simulink model "TosAeb"  (R2021a)
settings: Solver=FixedStepDiscrete  StartTime=0.0  StopTime=inf  FixedStep=0.01  RelTol=1e-3
undefined symbols (not defined in the file or in its folder): ACCModSts, AccSts1, AEB_ObjectMovingOrientation, AEB_ObjectMovingState, CN_BTCActive, CN_CurrentGearR, CN_CurrentGearR_DHT, CN_PTCActive, CN_VDCActive, CN_VSC_EspOff, CN_VSC_FCW_AEB_Error, CN_VSC_FCW_AEB_NoDispay, CN_VSC_FCW_AEB_PerfmDgdtn, CN_VSC_FCW_AEB_TempUnAvlbl, CN_VSC_VehStandstill, CNaccelAvoidMax, CNaccelAvoidMin, CNbrakePedalPress, CNcomfortStrLeftAccelMax, CNcomfortStrLeftAccelMin, CNcomfortStrRightAccelMax, CNcomfortStrRightAccelMin, CNDrvDoorOpen, CNDrvModeEco, CNDrvModeNorm, CNDrvModeOther, CNDrvModeSport, CNDrvSBRreminder, CNNull, CNobjInvalidID, CNPassDoorOpen, CNPassSBRreminder, CNPEBSAccelNegInf, CNPEBSAccelNull, CNPEBSTimeInf, CNPEBSTimeNull, CNSTOPMAX, CNSTOPNULL, CNsubjWidth, CNSwtOFF, CNSwtON, CNTTCMAX, CNTTCNULL, CNVehConfigAEBCycNone, CNVehConfigAEBNone, CNVehConfigAEBPedNone, CNVehConfigFCWNone, CNVehConfigJANone, CollisionSeverity, FALSE, FCWSnvty, JACycObjCalcInfoDefaultParams, JAPedObjCalcInfoDefaultParams, JAVehObjCalcInfoDefaultParams, latCycObjCalcInfoDefaultParams, latPedObjCalcInfoDefaultParams, longObjCalcInfoDefaultParams, ModSts, movingStatus, ObjectType ... (+1112)
libraries: simulink x3031, AEBSFunctionLib x50, Ta_Library x33, AEBSStateMachineLib x23, Mylibrary x12, simulink_extras x7, ObjSelect_lib x3, dspstat3 x2
(part 76 of 102)

== system TosAeb/AEB_Objsel/TA_Processor/PreProcessor/ObjectPrecessor/TAObjInfoCalc/For Each Subsystem/Info_Check1/Basic_Info_check/HeadAngle/If Action Subsystem1/HeadAngle_c/Subsystem2 ==
  Obj_vy : Inport
  Obj_Vx : Inport  Port=2
  Atan2 : Trigonometry  Operator=atan2
  Constant : Constant  Value=pi/2
  MapToAngularInterval : Ta_Library/MapToAngularInterval  SystemSampleTime=-1  VariantActivationTime=update diagram  Latency=0  AutoFrameSizeCalculation=off
  Obj_Head : Outport
  wires:
  Constant/1 -> MapToAngularInterval/2
  Obj_Vx/1 -> Atan2/2
  Obj_vy/1 -> Atan2/1
  Atan2/1 -> MapToAngularInterval/1
  MapToAngularInterval/1 -> Obj_Head/1

== system TosAeb/AEB_Objsel/TA_Processor/PreProcessor/ObjectPrecessor/TAObjInfoCalc/For Each Subsystem/Info_Check1/Basic_Info_check/QuaCorrect/quality_recrectionelse/Quality_else/Factor_Cal ==
  dy_flg : Inport
  dx_flg : Inport  Port=2
  vx_flg : Inport  Port=3
  vy_flg : Inport  Port=4
  Add : Sum  Inputs=++++
  Constant : Constant  Value=5  OutDataTypeStr=single
  Data Type Conversion{,1..3} (4 alike) : DataTypeConversion  OutDataTypeStr=single
  Product : Product
  factor : Outport
  wires:
  Data Type Conversion1/1 -> Add/2
  Data Type Conversion3/1 -> Add/4
  vy_flg/1 -> Data Type Conversion3/1
  dy_flg/1 -> Data Type Conversion/1
  Data Type Conversion/1 -> Add/1
  Add/1 -> Product/1
  dx_flg/1 -> Data Type Conversion1/1
  vx_flg/1 -> Data Type Conversion2/1
  Data Type Conversion2/1 -> Add/3
  Product/1 -> factor/1
  Constant/1 -> Product/2

== system TosAeb/AEB_Objsel/TA_Processor/PreProcessor/ObjectPrecessor/TAObjInfoCalc/For Each Subsystem/Info_Check1/Basic_Info_check/QuaCorrect/quality_recrectionelse/Quality_else/Quality_Out ==
  Fus_TargetQuality : Inport
  factor : Inport  Port=2
  Add : Sum  Inputs=+-
  Data Type Conversion : DataTypeConversion  OutDataTypeStr=single
  Data Type Conversion1 : DataTypeConversion  OutDataTypeStr=uint8
  Fus_ObjQuality : Outport
  wires:
  Data Type Conversion1/1 -> Fus_ObjQuality/1
  Add/1 -> Data Type Conversion1/1
  factor/1 -> Add/2
  Data Type Conversion/1 -> Add/1
  Fus_TargetQuality/1 -> Data Type Conversion/1

== system TosAeb/AEB_Objsel/TA_Processor/PreProcessor/ObjectPrecessor/TAObjInfoCalc/For Each Subsystem/Info_Check1/Basic_Info_check/VxCorrect/Subsystem3/Subsystem2/Subsystem ==
  Fus_ObjVx_Single : Inport
  Fus_ObjDx_Single : Inport  Port=2
  Subsystem : Subsystem
  VbyDx : Subsystem
  VbyVx : Subsystem
  DisLong_flg : Outport
  wires:
  Subsystem/1(DisLong_flg) -> DisLong_flg/1
  VbyVx/1(VbyVx) -> Subsystem/2(VbyVx)
  VbyDx/1(VbyDx) -> Subsystem/1(VbyDx)
  Fus_ObjVx_Single/1 -> VbyVx/1(Fus_ObjVx_Single)
  Fus_ObjDx_Single/1 -> VbyDx/1(Fus_ObjDx_Single)

== system TosAeb/AEB_Objsel/TA_Processor/PreProcessor/ObjectPrecessor/TAObjInfoCalc/For Each Subsystem/Info_Check1/Basic_Info_check/VxCorrect/Subsystem3/Subsystem2/Subsystem1 ==
  Veh_AEBFunState : Inport
  Compare To Constant : simulink/Logic and Bit Operations/Compare To Constant  relop===  const=0  OutDataTypeStr=boolean
  AEB_State : Outport
  wires:
  Compare To Constant/1 -> AEB_State/1
  Veh_AEBFunState/1 -> Compare To Constant/1

== system TosAeb/AEB_Objsel/TA_Processor/PreProcessor/ObjectPrecessor/TAObjInfoCalc/For Each Subsystem/Info_Check1/Basic_Info_check/VyCorrect/Subsystem3/Subsystem2/Subsystem ==
  Fus_ObjVy_Single : Inport
  Fus_ObjDy_Single : Inport  Port=2
  Subsystem : Subsystem
  VbyDy : Subsystem
  VbyVy : Subsystem
  DisLat_flg : Outport
  wires:
  Fus_ObjDy_Single/1 -> VbyDy/1(Fus_ObjDy_Single)
  Fus_ObjVy_Single/1 -> VbyVy/1(Fus_ObjVy_Single)
  VbyDy/1(VbyDy) -> Subsystem/1(VbyDy)
  VbyVy/1(VbyVy) -> Subsystem/2(VbyVy)
  Subsystem/1(DisLat_flg) -> DisLat_flg/1

== system TosAeb/AEB_Objsel/TA_Processor/PreProcessor/ObjectPrecessor/TAObjInfoCalc/For Each Subsystem/Info_Check1/Basic_Info_check/VyCorrect/Subsystem3/Subsystem2/Subsystem1 ==
  Veh_AEBFunState : Inport
  Compare To Constant : simulink/Logic and Bit Operations/Compare To Constant  relop===  const=1  OutDataTypeStr=boolean
  AEB_State : Outport
  wires:
  Veh_AEBFunState/1 -> Compare To Constant/1
  Compare To Constant/1 -> AEB_State/1

== system TosAeb/AEB_Objsel/TA_Processor/PreProcessor/ObjectPrecessor/TAObjInfoCalc/For Each Subsystem/Info_Check1/Basic_Info_check/VyCorrect/Subsystem3/Subsystem2/Subsystem2 ==
  Fus_TargetState : Inport
  Veh_YawRate : Inport  Port=2
  Abs : Abs
  Compare To Constant : simulink/Logic and Bit Operations/Compare To Constant  relop=<=  const=0.02  OutDataTypeStr=boolean
  Compare To Constant1 : simulink/Logic and Bit Operations/Compare To Constant  relop===  const=2  OutDataTypeStr=boolean
  Compare To Constant2 : simulink/Logic and Bit Operations/Compare To Constant  relop===  const=3  OutDataTypeStr=boolean
  Logical Operator : Logic  Operator=OR  AllPortsSameDT=off  OutDataTypeStr=boolean
  Logical Operator1 : Logic  AllPortsSameDT=off  OutDataTypeStr=boolean
  State_flg : Outport
  wires:
  Logical Operator1/1 -> State_flg/1
  Logical Operator/1 -> Logical Operator1/1
  Compare To Constant2/1 -> Logical Operator/2
  Compare To Constant1/1 -> Logical Operator/1
  Fus_TargetState/1 -> Compare To Constant1/1, Compare To Constant2/1
  Veh_YawRate/1 -> Abs/1
  Abs/1 -> Compare To Constant/1
  Compare To Constant/1 -> Logical Operator1/2

== system TosAeb/FcwAebJa_Step_sys/ALG_AEBSystem/ALG_AEBController/AEB_FuncImpManagementApp/FuncManeuArbCalc/brkFuncManeuArbCalc/axTgtReqValueCalc1/AebfullTriggerInfoCalc/AEBTriggerRelCalc/AEBTriggerInfo/AEBTriggerInfo/dxSecureCalc/dxSecureCalc ==
  subjVel : Inport
  vxPredicted : Inport  Port=2
  Abs1 : Abs
  dxSecure Lookup Table : Lookup_n-D  BreakpointsForDimension1=P_AEB_FIM_dxSecureX1_vEgo  BreakpointsForDimension2=P_AEB_FIM_dxSecureX2_vRel  Table=P_AEB_FIM_dxSecureY  InternalRulePriority=Speed  RndMeth=Simplest  InputSameDT=on
      settings: InputPortMap=u0,u1
  dxSecure : Outport
  wires:
  subjVel/1 -> dxSecure Lookup Table/1
  vxPredicted/1 -> Abs1/1
  dxSecure Lookup Table/1 -> dxSecure/1
  Abs1/1 -> dxSecure Lookup Table/2

== system TosAeb/FcwAebJa_Step_sys/ALG_AEBSystem/ALG_AEBController/AEB_FuncImpManagementApp/FuncManeuArbCalc/brkFuncManeuArbCalc/axTgtReqValueCalc1/AebfullTriggerInfoCalc/Subsystem/ObjectPredict/If Action Subsystem/dxPredictedCalc ==
  tPredicted : Inport
  dxFrontBumper : Inport  Port=2
  vx : Inport  Port=3
  ax : Inport  Port=4
  Add : Sum  Inputs=+++
  Constant : Constant  Value=0.5  OutDataTypeStr=single
  Constant1 : Constant  Value=CNNull
  Max : MinMax  Function=max  Inputs=2
  Product{,1..3} (4 alike) : Product
  dxFrontBumperPredicted : Outport
  wires:
  Max/1 -> dxFrontBumperPredicted/1
  Constant1/1 -> Max/2
  Product1/1 -> Product3/2
  tPredicted/1 -> Product/1, Product1/2, Product1/1
  dxFrontBumper/1 -> Add/1
  Add/1 -> Max/1
  Constant/1 -> Product2/2
  Product2/1 -> Product3/1
  ax/1 -> Product2/1
  Product/1 -> Add/3
  vx/1 -> Product/2
  Product3/1 -> Add/2

== system TosAeb/FcwAebJa_Step_sys/ALG_AEBSystem/ALG_AEBController/AEB_FuncImpManagementApp/FuncManeuArbCalc/brkFuncManeuArbCalc/axTgtReqValueCalc1/AebfullTriggerInfoCalc/Subsystem/ObjectPredict/If Action Subsystem/dxPredictedCalc1 ==
  ax : Inport
  tPredicted : Inport  Port=2
  vx : Inport  Port=3
  subjVel : Inport  Port=4
  Add1 : Sum
  Add2 : Sum
  Compare To Constant : simulink/Logic and Bit Operations/Compare To Constant  relop=<=  const=CNNull  OutDataTypeStr=boolean
  Constant : Constant  Value=CNNull
  Product3 : Product
  Subtract : Sum  Inputs=+-
  Switch : Switch  Criteria=u2 ~= 0
  vxAbsObjPredicted : Outport
  vxPredicted : Outport  Port=2
  wires:
  subjVel/1 -> Add2/2, Subtract/2
  Add1/1 -> Add2/1  "vxPredicted"
  Switch/1 -> Subtract/1, vxAbsObjPredicted/1
  Constant/1 -> Switch/1
  Compare To Constant/1 -> Switch/2
  Subtract/1 -> vxPredicted/1
  Add2/1 -> Compare To Constant/1, Switch/3
  vx/1 -> Add1/2
  Product3/1 -> Add1/1
  tPredicted/1 -> Product3/2
  ax/1 -> Product3/1

== system TosAeb/FcwAebJa_Step_sys/ALG_AEBSystem/ALG_AEBController/AEB_FuncImpManagementApp/FuncManeuArbCalc/brkFuncManeuArbCalc/axTgtReqValueCalc1/AebfullTriggerInfoCalc/Subsystem/ObjectPredict/If Action Subsystem/tPredictedCalc ==
  Constant : Constant  Value=P_AEB_FIM_ObjectPredictTimeMax
  Constant1 : Constant  Value=0  OutDataTypeStr=single
  MinMax1 : MinMax  Function=max  Inputs=2
  tPredicted : Outport
  wires:
  MinMax1/1 -> tPredicted/1  "AEB_FIM_ObjectPredictTime4Control"
  Constant/1 -> MinMax1/1
  Constant1/1 -> MinMax1/2

== system TosAeb/FcwAebJa_Step_sys/ALG_AEBSystem/ALG_AEBController/AEB_FuncImpManagementApp/FuncManeuArbCalc/brkFuncManeuArbCalc/axTgtReqValueCalc1/axTgtReqValueCalc4AebControl/JerkFuncDecCalc/JerkFuncDecCalc/JerkFuncDecCalc/JerkFuncDecCalc/JerkFuncDecCalc ==
  aErroraDesiredaTgtReqValue : Inport
  JerkFuncDecFactor : Inport  Port=2
  JerkFuncDecK1 : Inport  Port=3
  JerkFuncDecMax : Inport  Port=4
  Add : Sum
  Compare To Constant : simulink/Logic and Bit Operations/Compare To Constant  relop=<  const=CNNull  OutDataTypeStr=boolean
  Constant : Constant  Value=P_AEB_FIM_DecJerkStep4DecControl
  Constant1 : Constant  Value=CNNull
  Max : MinMax  Function=max  Inputs=2
  Product : Product
  Switch1 : Switch  Criteria=u2 ~= 0
  JerkFuncDec : Outport
  wires:
  Constant1/1 -> Switch1/3
  Product/1 -> Switch1/1
  JerkFuncDecFactor/1 -> Product/1
  Constant/1 -> Product/2
  aErroraDesiredaTgtReqValue/1 -> Compare To Constant/1
  Add/1 -> Max/1
  JerkFuncDecK1/1 -> Add/2
  Compare To Constant/1 -> Switch1/2
  Switch1/1 -> Add/1
  JerkFuncDecMax/1 -> Max/2
  Max/1 -> JerkFuncDec/1

== system TosAeb/FcwAebJa_Step_sys/ALG_AEBSystem/ALG_AEBController/AEB_FuncImpManagementApp/FuncManeuArbCalc/brkFuncManeuArbCalc/axTgtReqValueCalc1/axTgtReqValueCalc4AebControl/JerkFuncDecCalc/JerkFuncDecCalc/JerkFuncDecCalc/JerkFuncDecCalc/JerkFuncDecFactor ==
  note: 设计思路：
      | 若期望减速度小于最大减速度，
      | 则全速追踪
      | 若望减速度大于最大减速度，且小于阈值
      | 则放大系数，快速追踪
  note: 小误差放大，快速追踪目标值
  DeltaaDesiredaTgtReqValue : Inport
  aDesired : Inport  Port=2
  accelMin : Inport  Port=3
  1-D Lookup Table : Lookup_n-D  NumberOfTableDimensions=1  BreakpointsForDimension1=P_AEB_FIM_DecJerkFactorX_ErroraDesiredaTgt  Table=P_AEB_FIM_DecJerkFactorY  ExtrapMethod=Clip  InternalRulePriority=Speed  RndMeth=Simplest  InputSameDT=on
  Compare To Constant : simulink/Logic and Bit Operations/Compare To Constant  relop=>  const=CNNull  OutDataTypeStr=boolean
  Compare To Constant1 : simulink/Logic and Bit Operations/Compare To Constant  relop=>  const=P_AEB_FIM_aErroraDesiredaMaxThres4FastDec  OutDataTypeStr=boolean
  Constant : Constant  Value=250
  Constant1 : Constant  Value=P_AEB_FIM_FastDecFactorGain
  Product : Product
  Subtract1 : Sum  Inputs=+-
  Switch : Switch  Criteria=u2 ~= 0
  Switch1 : Switch  Criteria=u2 ~= 0
  JerkFuncDecFactor : Outport
  wires:
  DeltaaDesiredaTgtReqValue/1 -> 1-D Lookup Table/1
  accelMin/1 -> Subtract1/1
  aDesired/1 -> Subtract1/2
  Subtract1/1 -> Compare To Constant/1, Compare To Constant1/1  "DeltaaDesiredaMin"
  Switch1/1 -> Switch/3
  Constant/1 -> Switch/1
  Compare To Constant/1 -> Switch/2
  Compare To Constant1/1 -> Switch1/2
  1-D Lookup Table/1 -> Switch1/3, Product/2
  Constant1/1 -> Product/1
  Switch/1 -> JerkFuncDecFactor/1
  Product/1 -> Switch1/1

== system TosAeb/FcwAebJa_Step_sys/ALG_AEBSystem/ALG_AEBController/AEB_FuncImpManagementApp/FuncManeuArbCalc/brkFuncManeuArbCalc/axTgtReqValueCalc1/axTgtReqValueCalc4AebControl/JerkFuncDecCalc/JerkFuncDecCalc/JerkFuncDecCalc/JerkFuncDecCalc/aErroraDesiredaTgtReqValue ==
  axTgtReqValueK1 : Inport
  aDesired : Inport  Port=2
  Subtract : Sum  Inputs=+-
  aErroraDesiredaTgtReqValue : Outport
  wires:
  Subtract/1 -> aErroraDesiredaTgtReqValue/1
  aDesired/1 -> Subtract/1
  axTgtReqValueK1/1 -> Subtract/2

== system TosAeb/FcwAebJa_Step_sys/ALG_AEBSystem/ALG_AEBController/AEB_FuncImpManagementApp/FuncManeuArbCalc/brkFuncManeuArbCalc/axTgtReqValueCalc1/axTgtReqValueCalc4AebControl/aDesiredCalc/If Action Subsystem1/accelAvoidLowLvlBrkCalc/Situation1 ==
  dxEgoBrake : Inport
  vx : Inport  Port=2
  tObjStop : Inport  Port=3
  subjVel : Inport  Port=4
  vxAbsObj : Inport  Port=5
  axAbsObj : Inport  Port=6
  function : TriggerPort  TriggerType=function-call  VariantControl=Variant
  Bus Creator : BusCreator  Inputs=6
  Bus Selector : BusSelector  OutputSignals=dxEgoBrake,vx,tObjStop
  Bus Selector1 : BusSelector  OutputSignals=axAbsObj,vx,dxEgoBrake
  Bus Selector2 : BusSelector  OutputSignals=subjVel,vxAbsObj,axAbsObj,dxEgoBrake
  Case1 : Subsystem  TreatAsAtomicUnit=on
  Case2 : Subsystem  TreatAsAtomicUnit=on
  If : If  IfExpression=u1
  Merge : Merge
  collisionFirstFlgCalc : Subsystem
  accelAvoidLowLvlBrk : Outport
  wires:
  collisionFirstFlgCalc/1(collisionFirstFlg) -> If/1
  Bus Selector/3 -> collisionFirstFlgCalc/3(tObjStop)  "<tObjStop>"
  Bus Selector/2 -> collisionFirstFlgCalc/2(vx)  "<vx>"
  Bus Selector/1 -> collisionFirstFlgCalc/1(dxEgoBrake)  "<dxEgoBrake>"
  Bus Selector2/4 -> Case2/4(dxEgoBrake)  "<dxEgoBrake>"
  Bus Selector2/3 -> Case2/3(axAbsObj)  "<axAbsObj>"
  Bus Selector2/2 -> Case2/2(vxAbsObj)  "<vxAbsObj>"
  Bus Selector2/1 -> Case2/1(subjVel)  "<subjVel>"
  Bus Selector1/3 -> Case1/3(dxEgoBrake)  "<dxEgoBrake>"
  Bus Selector1/2 -> Case1/2(vx)  "<vx>"
  Bus Selector1/1 -> Case1/1(axAbsObj)  "<axAbsObj>"
  Bus Creator/1 -> Bus Selector/1, Bus Selector1/1, Bus Selector2/1
  vxAbsObj/1 -> Bus Creator/5  "vxAbsObj"
  axAbsObj/1 -> Bus Creator/6  "axAbsObj"
  dxEgoBrake/1 -> Bus Creator/1  "dxEgoBrake"
  subjVel/1 -> Bus Creator/4  "subjVel"
  vx/1 -> Bus Creator/2  "vx"
  tObjStop/1 -> Bus Creator/3  "tObjStop"
  [CASE2] -> Case2/Action
  If/2 -> [CASE2]
  Case2/1(accelAvoid) -> Merge/2
  Case1/1(accelAvoid) -> Merge/1
  Merge/1 -> accelAvoidLowLvlBrk/1
  If/1 -> Case1/Action

== system TosAeb/FcwAebJa_Step_sys/ALG_AEBSystem/ALG_AEBController/AEB_FuncImpManagementApp/FuncManeuArbCalc/brkFuncManeuArbCalc/axTgtReqValueCalc1/axTgtReqValueCalc4AebControl/aDesiredCalc/If Action Subsystem1/accelAvoidLowLvlBrkCalc/Situation2 ==
  axAbsObj : Inport
  vx : Inport  Port=2
  dxEgoBrake : Inport  Port=3
  function : TriggerPort  TriggerType=function-call  VariantControl=Variant
  Divide : Product  Inputs=*/
  Gain : Gain  Gain=2
  Math Function : Math  Operator=square  SignedPower=on  SaturateOnIntegerOverflow=on
  Subtract : Sum  Inputs=+-
  accelAvoidLowLvlBrk : Outport
  wires:
  Divide/1 -> Subtract/2
  Subtract/1 -> accelAvoidLowLvlBrk/1
  Gain/1 -> Divide/2
  dxEgoBrake/1 -> Gain/1
  Math Function/1 -> Divide/1
  vx/1 -> Math Function/1
  axAbsObj/1 -> Subtract/1

== system TosAeb/FcwAebJa_Step_sys/ALG_AEBSystem/ALG_AEBController/AEB_FuncImpManagementApp/FuncManeuArbCalc/brkFuncManeuArbCalc/axTgtReqValueCalc1/axTgtReqValueCalc4AebControl/aDesiredCalc/If Action Subsystem1/accelAvoidLowLvlBrkCalc/Situation3 ==
  note: aEgo^2
      | aAvoid = ----------------------------------
      | 		vObj^2
      | 		---------  - 2(dRel - dScure)
      | 		aObj
  subjVel : Inport
  vxAbsObj : Inport  Port=2
  axAbsObj : Inport  Port=3
  dxEgoBrake : Inport  Port=4
  function : TriggerPort  TriggerType=function-call  VariantControl=Variant
  Divide : Product  Inputs=*/
  Divide1 : Product  Inputs=*/
  Gain : Gain  Gain=2
  Math Function : Math  Operator=square  SignedPower=on  SaturateOnIntegerOverflow=on
  Math Function1 : Math  Operator=square  SignedPower=on  SaturateOnIntegerOverflow=on
  Subtract : Sum  Inputs=+-
  accelAvoidLowLvlBrk : Outport
  wires:
  Subtract/1 -> Divide1/2
  dxEgoBrake/1 -> Gain/1
  axAbsObj/1 -> Divide/2
  Divide/1 -> Subtract/1
  Math Function1/1 -> Divide1/1
  Divide1/1 -> accelAvoidLowLvlBrk/1
  Gain/1 -> Subtract/2
  Math Function/1 -> Divide/1
  subjVel/1 -> Math Function1/1
  vxAbsObj/1 -> Math Function/1

== system TosAeb/FcwAebJa_Step_sys/ALG_AEBSystem/ALG_AEBController/AEB_FuncImpManagementApp/FuncManeuArbCalc/brkFuncManeuArbCalc/axTgtReqValueCalc1/axTgtReqValueCalc4AebControl/aDesiredCalc/If Action Subsystem1/accelAvoidLowLvlBrkCalc/Situation4 ==
  function : TriggerPort  TriggerType=function-call  VariantControl=Variant
  Constant : Constant  Value=CNNull  VectorParams1D=off
  accelAvoidLowLvlBrk : Outport
  wires:
  Constant/1 -> accelAvoidLowLvlBrk/1

== system TosAeb/FcwAebJa_Step_sys/ALG_AEBSystem/ALG_AEBController/AEB_FuncImpManagementApp/FuncManeuArbCalc/brkFuncManeuArbCalc/axTgtReqValueCalc1/axTgtReqValueCalc4AebControl/aDesiredCalc/If Action Subsystem1/accelAvoidLowLvlBrkCalc/accelAvoidLowLvlBrkLimit ==
  accelAvoidLowLvlBrkNoLimit : Inport
  Saturation : Saturate  UpperLimit=CNNull  LowerLimit=CNaccelAvoidMin
      settings: AttributesFormatString=|
      | UpperLimit:%<UpperLimit>
      | LowerLimit:%<LowerLimit>
  accelAvoidLowLvlBrk : Outport
  wires:
  Saturation/1 -> accelAvoidLowLvlBrk/1
  accelAvoidLowLvlBrkNoLimit/1 -> Saturation/1

== system TosAeb/FcwAebJa_Step_sys/ALG_AEBSystem/ALG_AEBController/AEB_FuncImpManagementApp/FuncManeuArbCalc/brkFuncManeuArbCalc/axTgtReqValueCalc1/axTgtReqValueCalc4AebControl/aDesiredCalc/If Action Subsystem1/tObjStopCalc/StopTimeFlow/tStopFuncCalc ==
  velRel : Inport
  accelRel : Inport  Port=2
  tStopFuncCalc : TriggerPort  TriggerType=function-call  VariantControl=Variant
      settings: CopyFcn=Stateflow.SLINSF.SimulinkMan.removeBlockPreDeleteFcn  PreDeleteFcn=Stateflow.SLINSF.SimulinkMan.unsafePredeleteFcn
  Abs : Abs
  Abs1 : Abs
  Divide : Product  Inputs=*/
  temptStop : Outport
  wires:
  accelRel/1 -> Abs1/1
  Divide/1 -> temptStop/1
  Abs1/1 -> Divide/2
  Abs/1 -> Divide/1
  velRel/1 -> Abs/1

== system TosAeb/FcwAebJa_Step_sys/ALG_AEBSystem/ALG_AEBController/AEB_FuncImpManagementApp/FuncManeuArbCalc/brkFuncManeuArbCalc/axTgtReqValueCalc1/axTgtReqValueCalc4AebControl/axTgtReqValueCalc/axTgtReqValueFinal/axTgtReqValueCalc/GradientOpt ==
  JerkFuncInc : Inport
  JerkFuncDec : Inport  Port=2
  Compare To Constant : simulink/Logic and Bit Operations/Compare To Constant  relop=<  const=0  OutDataTypeStr=boolean
  Compare To Constant1 : simulink/Logic and Bit Operations/Compare To Constant  relop=>  const=0  OutDataTypeStr=boolean
  Constant : Constant  Value=64  OutDataTypeStr=single
  Constant1 : Constant  Value=-64  OutDataTypeStr=single
  Switch : Switch  Criteria=u2 ~= 0
  Switch1 : Switch  Criteria=u2 ~= 0
  JerkFuncIncOpt : Outport
  JerkFuncDecOpt : Outport  Port=2
  wires:
  Switch1/1 -> JerkFuncDecOpt/1
  Switch/1 -> JerkFuncIncOpt/1
  Constant1/1 -> Switch1/1
  Compare To Constant1/1 -> Switch1/2
  JerkFuncDec/1 -> Compare To Constant1/1, Switch1/3
  Constant/1 -> Switch/1
  JerkFuncInc/1 -> Compare To Constant/1, Switch/3
  Compare To Constant/1 -> Switch/2

== system TosAeb/FcwAebJa_Step_sys/ALG_AEBSystem/ALG_AEBController/AEB_FuncImpManagementApp/FuncManeuArbCalc/brkFuncManeuArbCalc/axTgtReqValueCalc1/axTgtReqValueCalc4AebControl/axTgtReqValueCalc/axTgtReqValueFinal/axTgtReqValueCalc/JerkTimeSlot ==
  JerkFuncInc : Inport
  JerkFuncDec : Inport  Port=2
  Ts : Inport  Port=3
  aDesired : Inport  Port=4
  axTgtReqValueK1 : Inport  Port=5
  Product : Product
  Product1 : Product
  Subtract : Sum  Inputs=+-
  JerkIncTimeSlot : Outport
  JerkDecTimeSlot : Outport  Port=2
  aErrorADesiredAxTgtReq : Outport  Port=3
  wires:
  Subtract/1 -> aErrorADesiredAxTgtReq/1
  axTgtReqValueK1/1 -> Subtract/2
  aDesired/1 -> Subtract/1
  Product1/1 -> JerkDecTimeSlot/1
  Product/1 -> JerkIncTimeSlot/1
  JerkFuncDec/1 -> Product1/2
  Ts/1 -> Product1/1, Product/2
  JerkFuncInc/1 -> Product/1

== system TosAeb/FcwAebJa_Step_sys/ALG_AEBSystem/ALG_AEBController/AEB_FuncImpManagementApp/FuncManeuArbCalc/brkFuncManeuArbCalc/axTgtReqValueCalc1/axTgtReqValueCalc4AebControl/axTgtReqValueCalc/axTgtReqValueFinal/axTgtReqValueCalc/axTgtReqValueCalc ==
  JerkIncTimeSlot : Inport
  JerkDecTimeSlot : Inport  Port=2
  aErrorADesiredAxTgtReq : Inport  Port=3
  accelMax : Inport  Port=4
  accelMin : Inport  Port=5
  axTgtReqValueK1 : Inport  Port=6
  aDesired : Inport  Port=7
  axTgtReqValueFlow : Stateflow chart  TreatAsAtomicUnit=on
  axTgtReqValue : Outport
  wires:
  axTgtReqValueFlow/1(axTgtReqValue) -> axTgtReqValue/1
  axTgtReqValueK1/1 -> axTgtReqValueFlow/7(axTgtReqValueK1)
  accelMin/1 -> axTgtReqValueFlow/6(accelMin)
  accelMax/1 -> axTgtReqValueFlow/5(accelMax)
  aDesired/1 -> axTgtReqValueFlow/4(accelAvoid)
  aErrorADesiredAxTgtReq/1 -> axTgtReqValueFlow/3(aErrorADesiredAxTgtReq)
  JerkDecTimeSlot/1 -> axTgtReqValueFlow/2(JerkDecTimeSlot)
  JerkIncTimeSlot/1 -> axTgtReqValueFlow/1(JerkIncTimeSlot)

== system TosAeb/FcwAebJa_Step_sys/ALG_AEBSystem/ALG_AEBController/AEB_FuncImpManagementApp/FuncManeuArbCalc/brkFuncManeuArbCalc/axTgtReqValueCalc1/axTgtReqValueCalc4AebControl/axTgtReqValueCalc/axTgtReqValueFinal1/axTgtReqValueCalc/GradientOpt ==
  JerkFuncInc : Inport
  JerkFuncDec : Inport  Port=2
  Compare To Constant : simulink/Logic and Bit Operations/Compare To Constant  relop=<  const=0  OutDataTypeStr=boolean
  Compare To Constant1 : simulink/Logic and Bit Operations/Compare To Constant  relop=>  const=0  OutDataTypeStr=boolean
  Constant : Constant  Value=64  OutDataTypeStr=single
  Constant1 : Constant  Value=-64  OutDataTypeStr=single
  Switch : Switch  Criteria=u2 ~= 0
  Switch1 : Switch  Criteria=u2 ~= 0
  JerkFuncIncOpt : Outport
  JerkFuncDecOpt : Outport  Port=2
  wires:
  Switch1/1 -> JerkFuncDecOpt/1
  Switch/1 -> JerkFuncIncOpt/1
  Constant1/1 -> Switch1/1
  Compare To Constant1/1 -> Switch1/2
  JerkFuncDec/1 -> Compare To Constant1/1, Switch1/3
  Constant/1 -> Switch/1
  JerkFuncInc/1 -> Compare To Constant/1, Switch/3
  Compare To Constant/1 -> Switch/2

== system TosAeb/FcwAebJa_Step_sys/ALG_AEBSystem/ALG_AEBController/AEB_FuncImpManagementApp/FuncManeuArbCalc/brkFuncManeuArbCalc/axTgtReqValueCalc1/axTgtReqValueCalc4AebControl/axTgtReqValueCalc/axTgtReqValueFinal1/axTgtReqValueCalc/JerkTimeSlot ==
  JerkFuncInc : Inport
  JerkFuncDec : Inport  Port=2
  Ts : Inport  Port=3
  aDesired : Inport  Port=4
  axTgtReqValueK1 : Inport  Port=5
  Product : Product
  Product1 : Product
  Subtract : Sum  Inputs=+-
  JerkIncTimeSlot : Outport
  JerkDecTimeSlot : Outport  Port=2
  aErrorADesiredAxTgtReq : Outport  Port=3
  wires:
  Subtract/1 -> aErrorADesiredAxTgtReq/1
  axTgtReqValueK1/1 -> Subtract/2
  aDesired/1 -> Subtract/1
  Product1/1 -> JerkDecTimeSlot/1
  Product/1 -> JerkIncTimeSlot/1
  JerkFuncDec/1 -> Product1/2
  Ts/1 -> Product1/1, Product/2
  JerkFuncInc/1 -> Product/1

== system TosAeb/FcwAebJa_Step_sys/ALG_AEBSystem/ALG_AEBController/AEB_FuncImpManagementApp/FuncManeuArbCalc/brkFuncManeuArbCalc/axTgtReqValueCalc1/axTgtReqValueCalc4AebControl/axTgtReqValueCalc/axTgtReqValueFinal1/axTgtReqValueCalc/axTgtReqValueCalc ==
  JerkIncTimeSlot : Inport
  JerkDecTimeSlot : Inport  Port=2
  aErrorADesiredAxTgtReq : Inport  Port=3
  accelMax : Inport  Port=4
  accelMin : Inport  Port=5
  axTgtReqValueK1 : Inport  Port=6
  aDesired : Inport  Port=7
  axTgtReqValueFlow : Stateflow chart  TreatAsAtomicUnit=on
  axTgtReqValue : Outport
  wires:
  axTgtReqValueFlow/1(axTgtReqValue) -> axTgtReqValue/1
  axTgtReqValueK1/1 -> axTgtReqValueFlow/7(axTgtReqValueK1)
  accelMin/1 -> axTgtReqValueFlow/6(accelMin)
  accelMax/1 -> axTgtReqValueFlow/5(accelMax)
  aDesired/1 -> axTgtReqValueFlow/4(accelAvoid)
  aErrorADesiredAxTgtReq/1 -> axTgtReqValueFlow/3(aErrorADesiredAxTgtReq)
  JerkDecTimeSlot/1 -> axTgtReqValueFlow/2(JerkDecTimeSlot)
  JerkIncTimeSlot/1 -> axTgtReqValueFlow/1(JerkIncTimeSlot)

== system TosAeb/FcwAebJa_Step_sys/ALG_AEBSystem/ALG_AEBController/AEB_JaObjCalcApp/JAPedTgtInfoCalc/JAPedObjCalcInfoCalc/JAPedObjInfoCalc/ObjCrossFlgCal/CollisionProbCalc/Subsystem/ObjBoundingBox/ObjLLCoordinate ==
  inputSignals : Inport
  Add : Sum  Inputs=+-  OutDataTypeStr=single
  Add1 : Sum  OutDataTypeStr=single
  Bus Selector : BusSelector  OutputSignals=dxPreRefPointObj,ObjWidthCorr,sincosCoef.sinCoef
  Bus Selector1 : BusSelector  OutputSignals=dyPreRefPointObj,ObjWidthCorr,sincosCoef.cosCoef
  Gain : Gain  Gain=0.5  OutDataTypeStr=single
  Gain1 : Gain  Gain=0.5  OutDataTypeStr=single
  Product1 : Product  OutDataTypeStr=single
  Product3 : Product  OutDataTypeStr=single
  dxPre_LLObj : Outport
  dyPreLLObj : Outport  Port=2
  wires:
  Bus Selector1/1 -> Add1/1  "<dyPreRefPointObj>"
  Bus Selector1/3 -> Product3/2  "<cosCoef>"
  Bus Selector1/2 -> Product3/1  "<ObjWidthCorr>"
  Product3/1 -> Gain/1
  Add1/1 -> dyPreLLObj/1
  Add/1 -> dxPre_LLObj/1
  Bus Selector/1 -> Add/1  "<dxPreRefPointObj>"
  Product1/1 -> Gain1/1
  Bus Selector/3 -> Product1/2  "<sinCoef>"
  Bus Selector/2 -> Product1/1  "<ObjWidthCorr>"
  inputSignals/1 -> Bus Selector1/1, Bus Selector/1
  Gain/1 -> Add1/2
  Gain1/1 -> Add/2

== system TosAeb/FcwAebJa_Step_sys/ALG_AEBSystem/ALG_AEBController/AEB_JaObjCalcApp/JAPedTgtInfoCalc/JAPedObjCalcInfoCalc/JAPedObjInfoCalc/ObjCrossFlgCal/CollisionProbCalc/Subsystem/ObjBoundingBox/ObjLUCoordinate ==
  inputSignals : Inport
  Add : Sum  Inputs=++-  OutDataTypeStr=single
  Add1 : Sum  Inputs=+++  OutDataTypeStr=single
  Bus Selector : BusSelector  OutputSignals=dxPreRefPointObj,ObjLengthCorr,sincosCoef.cosCoef,ObjWidthCorr,sincosCoef.sinCoef
  Bus Selector1 : BusSelector  OutputSignals=dyPreRefPointObj,ObjLengthCorr,sincosCoef.sinCoef,ObjWidthCorr,sincosCoef.cosCoef
  Gain : Gain  Gain=0.5  OutDataTypeStr=single
  Gain1 : Gain  Gain=0.5  OutDataTypeStr=single
  Product{,1..3} (4 alike) : Product  OutDataTypeStr=single
  dxPreLUObj : Outport
  dyPreLUobj : Outport  Port=2
  wires:
  inputSignals/1 -> Bus Selector1/1, Bus Selector/1
  Bus Selector/2 -> Product/1  "<ObjLengthCorr>"
  Bus Selector/3 -> Product/2  "<cosCoef>"
  Bus Selector/4 -> Product1/1  "<ObjWidthCorr>"
  Bus Selector/5 -> Product1/2  "<sinCoef>"
  Product1/1 -> Gain1/1
  Bus Selector/1 -> Add/1  "<dxPreRefPointObj>"
  Product/1 -> Add/2
  Add/1 -> dxPreLUObj/1
  Add1/1 -> dyPreLUobj/1
  Product2/1 -> Add1/2
  Product3/1 -> Gain/1
  Bus Selector1/2 -> Product2/1  "<ObjLengthCorr>"
  Bus Selector1/4 -> Product3/1  "<ObjWidthCorr>"
  Bus Selector1/3 -> Product2/2  "<sinCoef>"
  Bus Selector1/5 -> Product3/2  "<cosCoef>"
  Bus Selector1/1 -> Add1/1  "<dyPreRefPointObj>"
  Gain/1 -> Add1/3
  Gain1/1 -> Add/3

== system TosAeb/FcwAebJa_Step_sys/ALG_AEBSystem/ALG_AEBController/AEB_JaObjCalcApp/JAPedTgtInfoCalc/JAPedObjCalcInfoCalc/JAPedObjInfoCalc/ObjCrossFlgCal/CollisionProbCalc/Subsystem/ObjBoundingBox/ObjRLCoordinate ==
  inputSignals : Inport
  Add : Sum  OutDataTypeStr=single
  Add1 : Sum  Inputs=+-  OutDataTypeStr=single
  Bus Selector : BusSelector  OutputSignals=dxPreRefPointObj,ObjWidthCorr,sincosCoef.sinCoef
  Bus Selector1 : BusSelector  OutputSignals=dyPreRefPointObj,ObjWidthCorr,sincosCoef.cosCoef
  Gain : Gain  Gain=0.5  OutDataTypeStr=single
  Gain1 : Gain  Gain=0.5  OutDataTypeStr=single
  Product1 : Product  OutDataTypeStr=single
  Product3 : Product  OutDataTypeStr=single
  dxPreRLObj : Outport
  dyPreRLObj : Outport  Port=2
  wires:
  Bus Selector1/1 -> Add1/1  "<dyPreRefPointObj>"
  Bus Selector1/3 -> Product3/2  "<cosCoef>"
  Bus Selector1/2 -> Product3/1  "<ObjWidthCorr>"
  Product3/1 -> Gain1/1
  Add1/1 -> dyPreRLObj/1
  Add/1 -> dxPreRLObj/1
  Bus Selector/1 -> Add/1  "<dxPreRefPointObj>"
  Product1/1 -> Gain/1
  Bus Selector/3 -> Product1/2  "<sinCoef>"
  Bus Selector/2 -> Product1/1  "<ObjWidthCorr>"
  inputSignals/1 -> Bus Selector1/1, Bus Selector/1
  Gain/1 -> Add/2
  Gain1/1 -> Add1/2

== system TosAeb/FcwAebJa_Step_sys/ALG_AEBSystem/ALG_AEBController/AEB_JaObjCalcApp/JAPedTgtInfoCalc/JAPedObjCalcInfoCalc/JAPedObjInfoCalc/ObjCrossFlgCal/CollisionProbCalc/Subsystem/ObjBoundingBox/ObjRUCoordinate ==
  inputSignals : Inport
  Add : Sum  Inputs=+++  OutDataTypeStr=single
  Add1 : Sum  Inputs=++-  OutDataTypeStr=single
  Bus Selector : BusSelector  OutputSignals=dxPreRefPointObj,ObjLengthCorr,sincosCoef.cosCoef,ObjWidthCorr,sincosCoef.sinCoef
  Bus Selector1 : BusSelector  OutputSignals=dyPreRefPointObj,ObjLengthCorr,sincosCoef.sinCoef,ObjWidthCorr,sincosCoef.cosCoef
  Gain : Gain  Gain=0.5  OutDataTypeStr=single
  Gain1 : Gain  Gain=0.5  OutDataTypeStr=single
  Product{,1..3} (4 alike) : Product  OutDataTypeStr=single
  dxPreRUObj : Outport
  dyPreRUObj : Outport  Port=2
  wires:
  inputSignals/1 -> Bus Selector1/1, Bus Selector/1
  Bus Selector/2 -> Product/1  "<ObjLengthCorr>"
  Bus Selector/3 -> Product/2  "<cosCoef>"
  Bus Selector/4 -> Product1/1  "<ObjWidthCorr>"
  Bus Selector/5 -> Product1/2  "<sinCoef>"
  Product1/1 -> Gain1/1
  Bus Selector/1 -> Add/1  "<dxPreRefPointObj>"
  Product/1 -> Add/2
  Add/1 -> dxPreRUObj/1
  Add1/1 -> dyPreRUObj/1
  Product2/1 -> Add1/2
  Product3/1 -> Gain/1
  Bus Selector1/2 -> Product2/1  "<ObjLengthCorr>"
  Bus Selector1/4 -> Product3/1  "<ObjWidthCorr>"
  Bus Selector1/3 -> Product2/2  "<sinCoef>"
  Bus Selector1/5 -> Product3/2  "<cosCoef>"
  Bus Selector1/1 -> Add1/1  "<dyPreRefPointObj>"
  Gain/1 -> Add1/3
  Gain1/1 -> Add/3

== system TosAeb/FcwAebJa_Step_sys/ALG_AEBSystem/ALG_AEBController/AEB_JaObjCalcApp/JAPedTgtInfoCalc/JAPedObjCalcInfoCalc/JAPedObjInfoCalc/ObjCrossFlgCal/CollisionProbCalc/Subsystem/PreCrossFlgCalcFlow/VectorCrossAlgorithm/If Action Subsystem ==
  Action Port : ActionPort  ActionPortLabel=if(u1)
  Constant : Constant  Value=0  OutDataTypeStr=boolean
  CrossFlg : Outport
  wires:
  Constant/1 -> CrossFlg/1

== system TosAeb/FcwAebJa_Step_sys/ALG_AEBSystem/ALG_AEBController/AEB_JaObjCalcApp/JAPedTgtInfoCalc/JAPedObjCalcInfoCalc/JAPedObjInfoCalc/ObjCrossFlgCal/CollisionProbCalc/Subsystem/PreCrossFlgCalcFlow/VectorCrossAlgorithm/If Action Subsystem1 ==
  vectorMulit : Inport
  Coordinations : Inport  Port=2
  Action Port : ActionPort  ActionPortLabel=else
  Bus Selector : BusSelector  OutputSignals=m2,m1,y1,n1,n2,x1
  Bus Selector1 : BusSelector  OutputSignals=x2,x1,y1,n1,y2,m1
  Subsystem : Subsystem
  t : Subsystem
  u : Subsystem
  CrossFlg : Outport
  wires:
  Coordinations/1 -> Bus Selector1/1, Bus Selector/1
  Bus Selector/2 -> t/2(m1)  "<m1>"
  Bus Selector/3 -> t/3(y1)  "<y1>"
  Bus Selector/1 -> t/1(m2)  "<m2>"
  Bus Selector/4 -> t/4(n1)  "<n1>"
  Bus Selector/5 -> t/5(n2)  "<n2>"
  Bus Selector/6 -> t/6(x1)  "<x1>"
  Bus Selector1/3 -> u/3(y1)  "<y1>"
  Bus Selector1/2 -> u/2(x1)  "<x1>"
  Bus Selector1/4 -> u/4(n1)  "<n1>"
  Bus Selector1/1 -> u/1(x2)  "<x2>"
  Bus Selector1/5 -> u/5(y2)  "<y2>"
  Bus Selector1/6 -> u/6(m1)  "<m1>"
  t/1(temp_t) -> Subsystem/1(temp_u)
  u/1(temp_u) -> Subsystem/2(temp_t)
  Subsystem/1(CrossFlg) -> CrossFlg/1
  vectorMulit/1 -> Subsystem/3(vectorMulit)

== system TosAeb/FcwAebJa_Step_sys/ALG_AEBSystem/ALG_AEBController/AEB_JaObjCalcApp/JAPedTgtInfoCalc/JAPedObjCalcInfoCalc/JAPedObjInfoCalc/ObjCrossFlgCal/CollisionProbCalc/Subsystem/PreCrossFlgCalcFlow/VectorCrossAlgorithm/VectorMulit ==
  x1 : Inport  OutDataTypeStr=single
  x2 : Inport  Port=2  OutDataTypeStr=single
  n2 : Inport  Port=3  OutDataTypeStr=single
  n1 : Inport  Port=4  OutDataTypeStr=single
  y2 : Inport  Port=5  OutDataTypeStr=single
  y1 : Inport  Port=6  OutDataTypeStr=single
  m2 : Inport  Port=7  OutDataTypeStr=single
  m1 : Inport  Port=8  OutDataTypeStr=single
  Product : Product  OutDataTypeStr=single
  Product1 : Product  OutDataTypeStr=single
  Subtract{1..4} (4 alike) : Sum  Inputs=+-  OutDataTypeStr=single
  Subtract5 : Sum  Inputs=+-
  vectorMulit : Outport
  wires:
  x1/1 -> Subtract2/2
  x2/1 -> Subtract2/1
  Subtract2/1 -> Product/1
  Subtract3/1 -> Product/2
  y2/1 -> Subtract4/1
  y1/1 -> Subtract4/2
  m2/1 -> Subtract1/1
  m1/1 -> Subtract1/2
  Subtract4/1 -> Product1/1
  Subtract1/1 -> Product1/2
  Product/1 -> Subtract5/1
  Product1/1 -> Subtract5/2
  Subtract5/1 -> vectorMulit/1
  n2/1 -> Subtract3/1
  n1/1 -> Subtract3/2

== system TosAeb/FcwAebJa_Step_sys/ALG_AEBSystem/ALG_AEBController/AEB_JaObjCalcApp/JAPedTgtInfoCalc/JAPedObjCalcInfoCalc/JAPedObjInfoCalc/ObjCrossFlgCal/CollisionProbCalc/Subsystem/SubjBoundingBox/SubjLLCoordinate ==
  inputSignals : Inport
  Add2 : Sum  Inputs=+--  OutDataTypeStr=single
  Add3 : Sum  Inputs=+-+  OutDataTypeStr=single
  Bus Selector2 : BusSelector  OutputSignals=dxPreRefPoint,dxRearBumperToRearAxis,sincosCoef.cosCoef,subjWidth,sincosCoef.sinCoef
  Bus Selector3 : BusSelector  OutputSignals=dyPreRefPoint,dxRearBumperToRearAxis,sincosCoef.sinCoef,subjWidth,sincosCoef.cosCoef
  Gain2 : Gain  Gain=0.5  OutDataTypeStr=single
  Gain3 : Gain  Gain=0.5  OutDataTypeStr=single
  Product{4..7} (4 alike) : Product  OutDataTypeStr=single
  dxPreLL : Outport
  dyPreLL : Outport  Port=2
  wires:
  Gain3/1 -> Add3/3
  Bus Selector3/3 -> Product6/2  "<sinCoef>"
  Add3/1 -> dyPreLL/1
  Product7/1 -> Gain3/1
  Product6/1 -> Add3/2
  Product4/1 -> Add2/2
  Bus Selector3/5 -> Product7/2  "<cosCoef>"
  Product5/1 -> Gain2/1
  Gain2/1 -> Add2/3
  Add2/1 -> dxPreLL/1
  Bus Selector3/4 -> Product7/1  "<subjWidth>"
  Bus Selector3/2 -> Product6/1  "<dxRearBumperToRearAxis>"
  Bus Selector3/1 -> Add3/1  "<dyPreRefPoint>"
  inputSignals/1 -> Bus Selector3/1, Bus Selector2/1
  Bus Selector2/4 -> Product5/1  "<subjWidth>"
  Bus Selector2/2 -> Product4/1  "<dxRearBumperToRearAxis>"
  Bus Selector2/3 -> Product4/2  "<cosCoef>"
  Bus Selector2/1 -> Add2/1  "<dxPreRefPoint>"
  Bus Selector2/5 -> Product5/2  "<sinCoef>"

== system TosAeb/FcwAebJa_Step_sys/ALG_AEBSystem/ALG_AEBController/AEB_JaObjCalcApp/JAPedTgtInfoCalc/JAPedObjCalcInfoCalc/JAPedObjInfoCalc/ObjCrossFlgCal/CollisionProbCalc/Subsystem/SubjBoundingBox/SubjLUCoordinate ==
  inputSignals : Inport
  Add : Sum  Inputs=++-  OutDataTypeStr=single
  Add1 : Sum  Inputs=+++  OutDataTypeStr=single
  Bus Selector : BusSelector  OutputSignals=dxPreRefPoint,dxFrontBumperToRearAxis,sincosCoef.cosCoef,subjWidth,sincosCoef.sinCoef
  Bus Selector1 : BusSelector  OutputSignals=dyPreRefPoint,dxFrontBumperToRearAxis,sincosCoef.sinCoef,subjWidth,sincosCoef.cosCoef
  Gain : Gain  Gain=0.5  OutDataTypeStr=single
  Gain1 : Gain  Gain=0.5  OutDataTypeStr=single
  Product{,1..3} (4 alike) : Product  OutDataTypeStr=single
  dxPreLU : Outport
  dyPreLU : Outport  Port=2
  wires:
  Bus Selector/4 -> Product1/1  "<subjWidth>"
  Product3/1 -> Gain1/1
  Add/1 -> dxPreLU/1
  Bus Selector1/1 -> Add1/1  "<dyPreRefPoint>"
  Gain1/1 -> Add1/3
  Bus Selector1/4 -> Product3/1  "<subjWidth>"
  Product2/1 -> Add1/2
  Bus Selector/3 -> Product/2  "<cosCoef>"
  Add1/1 -> dyPreLU/1
  Bus Selector/5 -> Product1/2  "<sinCoef>"
  Bus Selector/2 -> Product/1  "<dxFrontBumperToRearAxis>"
  Bus Selector1/2 -> Product2/1  "<dxFrontBumperToRearAxis>"
  inputSignals/1 -> Bus Selector1/1, Bus Selector/1
  Bus Selector1/5 -> Product3/2  "<cosCoef>"
  Bus Selector/1 -> Add/1  "<dxPreRefPoint>"
  Gain/1 -> Add/3
  Product1/1 -> Gain/1
  Product/1 -> Add/2
  Bus Selector1/3 -> Product2/2  "<sinCoef>"

== system TosAeb/FcwAebJa_Step_sys/ALG_AEBSystem/ALG_AEBController/AEB_JaObjCalcApp/JAPedTgtInfoCalc/JAPedObjCalcInfoCalc/JAPedObjInfoCalc/ObjCrossFlgCal/CollisionProbCalc/Subsystem/SubjBoundingBox/SubjRLCoordinate ==
  inputSignals : Inport
  Add6 : Sum  Inputs=+-+  OutDataTypeStr=single
  Add7 : Sum  Inputs=+--  OutDataTypeStr=single
  Bus Selector6 : BusSelector  OutputSignals=dxPreRefPoint,dxRearBumperToRearAxis,sincosCoef.cosCoef,subjWidth,sincosCoef.sinCoef
  Bus Selector7 : BusSelector  OutputSignals=dyPreRefPoint,dxRearBumperToRearAxis,sincosCoef.sinCoef,subjWidth,sincosCoef.cosCoef
  Gain6 : Gain  Gain=0.5  OutDataTypeStr=single
  Gain7 : Gain  Gain=0.5  OutDataTypeStr=single
  Product{12..15} (4 alike) : Product  OutDataTypeStr=single
  dxPreRL : Outport
  dyPreRL : Outport  Port=2
  wires:
  Product12/1 -> Add6/2
  Gain6/1 -> Add6/3
  Product13/1 -> Gain6/1
  Add6/1 -> dxPreRL/1
  Bus Selector6/4 -> Product13/1  "<subjWidth>"
  Bus Selector6/5 -> Product13/2  "<sinCoef>"
  Bus Selector6/1 -> Add6/1  "<dxPreRefPoint>"
  Bus Selector6/2 -> Product12/1  "<dxRearBumperToRearAxis>"
  Bus Selector6/3 -> Product12/2  "<cosCoef>"
  inputSignals/1 -> Bus Selector7/1, Bus Selector6/1
  Add7/1 -> dyPreRL/1
  Product14/1 -> Add7/2
  Gain7/1 -> Add7/3
  Bus Selector7/1 -> Add7/1  "<dyPreRefPoint>"
  Bus Selector7/2 -> Product14/1  "<dxRearBumperToRearAxis>"
  Bus Selector7/4 -> Product15/1  "<subjWidth>"
  Product15/1 -> Gain7/1
  Bus Selector7/5 -> Product15/2  "<cosCoef>"
  Bus Selector7/3 -> Product14/2  "<sinCoef>"

== system TosAeb/FcwAebJa_Step_sys/ALG_AEBSystem/ALG_AEBController/AEB_JaObjCalcApp/JAPedTgtInfoCalc/JAPedObjCalcInfoCalc/JAPedObjInfoCalc/ObjCrossFlgCal/CollisionProbCalc/Subsystem/SubjBoundingBox/SubjRUCoordinate ==
  inputSignals : Inport
  Add4 : Sum  Inputs=+++  OutDataTypeStr=single
  Add5 : Sum  Inputs=++-  OutDataTypeStr=single
  Bus Selector4 : BusSelector  OutputSignals=dxPreRefPoint,dxFrontBumperToRearAxis,sincosCoef.cosCoef,subjWidth,sincosCoef.sinCoef
  Bus Selector5 : BusSelector  OutputSignals=dyPreRefPoint,dxFrontBumperToRearAxis,sincosCoef.sinCoef,subjWidth,sincosCoef.cosCoef
  Gain4 : Gain  Gain=0.5  OutDataTypeStr=single
  Gain5 : Gain  Gain=0.5  OutDataTypeStr=single
  Product{8..11} (4 alike) : Product  OutDataTypeStr=single
  dxPreRU : Outport
  dyPreRU : Outport  Port=2
  wires:
  Bus Selector4/4 -> Product9/1  "<subjWidth>"
  Bus Selector4/5 -> Product9/2  "<sinCoef>"
  Bus Selector4/3 -> Product8/2  "<cosCoef>"
  Bus Selector4/1 -> Add4/1  "<dxPreRefPoint>"
  inputSignals/1 -> Bus Selector5/1, Bus Selector4/1
  Bus Selector4/2 -> Product8/1  "<dxFrontBumperToRearAxis>"
  Product9/1 -> Gain4/1
  Bus Selector5/4 -> Product11/1  "<subjWidth>"
  Product10/1 -> Add5/2
  Product11/1 -> Gain5/1
  Add5/1 -> dyPreRU/1
  Gain5/1 -> Add5/3
  Bus Selector5/2 -> Product10/1  "<dxFrontBumperToRearAxis>"
  Bus Selector5/3 -> Product10/2  "<sinCoef>"
  Bus Selector5/5 -> Product11/2  "<cosCoef>"
  Add4/1 -> dxPreRU/1
  Gain4/1 -> Add4/3
  Product8/1 -> Add4/2
  Bus Selector5/1 -> Add5/1  "<dyPreRefPoint>"

== system TosAeb/FcwAebJa_Step_sys/ALG_AEBSystem/ALG_AEBController/AEB_JaObjCalcApp/JAVehTgtInfoCalc/AEBJAVehObjCalc/JAVehObjInfoCalc/ObjCrossFlgCal/CollisionProbCalc/Subsystem/ObjBoundingBoxRear/ObjLLCoordinate ==
  inputSignals : Inport
  Add : Sum  Inputs=+-  OutDataTypeStr=single
  Add1 : Sum  OutDataTypeStr=single
  Bus Selector : BusSelector  OutputSignals=dxPreRefPointObj,ObjWidthCorr,sincosCoef.sinCoef
  Bus Selector1 : BusSelector  OutputSignals=dyPreRefPointObj,ObjWidthCorr,sincosCoef.cosCoef
  Gain : Gain  Gain=0.5  OutDataTypeStr=single
  Gain1 : Gain  Gain=0.5  OutDataTypeStr=single
  Product1 : Product  OutDataTypeStr=single
  Product3 : Product  OutDataTypeStr=single
  dxPre_LLObj : Outport
  dyPreLLObj : Outport  Port=2
  wires:
  Bus Selector1/1 -> Add1/1  "<dyPreRefPointObj>"
  Bus Selector1/3 -> Product3/2  "<cosCoef>"
  Bus Selector1/2 -> Product3/1  "<ObjWidthCorr>"
  Product3/1 -> Gain/1
  Add1/1 -> dyPreLLObj/1
  Add/1 -> dxPre_LLObj/1
  Bus Selector/1 -> Add/1  "<dxPreRefPointObj>"
  Product1/1 -> Gain1/1
  Bus Selector/3 -> Product1/2  "<sinCoef>"
  Bus Selector/2 -> Product1/1  "<ObjWidthCorr>"
  inputSignals/1 -> Bus Selector1/1, Bus Selector/1
  Gain/1 -> Add1/2
  Gain1/1 -> Add/2

== system TosAeb/FcwAebJa_Step_sys/ALG_AEBSystem/ALG_AEBController/AEB_JaObjCalcApp/JAVehTgtInfoCalc/AEBJAVehObjCalc/JAVehObjInfoCalc/ObjCrossFlgCal/CollisionProbCalc/Subsystem/ObjBoundingBoxRear/ObjLUCoordinate ==
  inputSignals : Inport
  Add : Sum  Inputs=++-  OutDataTypeStr=single
  Add1 : Sum  Inputs=+++  OutDataTypeStr=single
  Bus Selector : BusSelector  OutputSignals=dxPreRefPointObj,ObjLengthCorr,sincosCoef.cosCoef,ObjWidthCorr,sincosCoef.sinCoef
  Bus Selector1 : BusSelector  OutputSignals=dyPreRefPointObj,ObjLengthCorr,sincosCoef.sinCoef,ObjWidthCorr,sincosCoef.cosCoef
  Gain : Gain  Gain=0.5  OutDataTypeStr=single
  Gain1 : Gain  Gain=0.5  OutDataTypeStr=single
  Product{,1..3} (4 alike) : Product  OutDataTypeStr=single
  dxPreLUObj : Outport
  dyPreLUobj : Outport  Port=2
  wires:
  inputSignals/1 -> Bus Selector1/1, Bus Selector/1
  Bus Selector/2 -> Product/1  "<ObjLengthCorr>"
  Bus Selector/3 -> Product/2  "<cosCoef>"
  Bus Selector/4 -> Product1/1  "<ObjWidthCorr>"
  Bus Selector/5 -> Product1/2  "<sinCoef>"
  Product1/1 -> Gain1/1
  Bus Selector/1 -> Add/1  "<dxPreRefPointObj>"
  Product/1 -> Add/2
  Add/1 -> dxPreLUObj/1
  Add1/1 -> dyPreLUobj/1
  Product2/1 -> Add1/2
  Product3/1 -> Gain/1
  Bus Selector1/2 -> Product2/1  "<ObjLengthCorr>"
  Bus Selector1/4 -> Product3/1  "<ObjWidthCorr>"
  Bus Selector1/3 -> Product2/2  "<sinCoef>"
  Bus Selector1/5 -> Product3/2  "<cosCoef>"
  Bus Selector1/1 -> Add1/1  "<dyPreRefPointObj>"
  Gain/1 -> Add1/3
  Gain1/1 -> Add/3

== system TosAeb/FcwAebJa_Step_sys/ALG_AEBSystem/ALG_AEBController/AEB_JaObjCalcApp/JAVehTgtInfoCalc/AEBJAVehObjCalc/JAVehObjInfoCalc/ObjCrossFlgCal/CollisionProbCalc/Subsystem/ObjBoundingBoxRear/ObjRLCoordinate ==
  inputSignals : Inport
  Add : Sum  OutDataTypeStr=single
  Add1 : Sum  Inputs=+-  OutDataTypeStr=single
  Bus Selector : BusSelector  OutputSignals=dxPreRefPointObj,ObjWidthCorr,sincosCoef.sinCoef
  Bus Selector1 : BusSelector  OutputSignals=dyPreRefPointObj,ObjWidthCorr,sincosCoef.cosCoef
  Gain : Gain  Gain=0.5  OutDataTypeStr=single
  Gain1 : Gain  Gain=0.5  OutDataTypeStr=single
  Product1 : Product  OutDataTypeStr=single
  Product3 : Product  OutDataTypeStr=single
  dxPreRLObj : Outport
  dyPreRLObj : Outport  Port=2
  wires:
  Bus Selector1/1 -> Add1/1  "<dyPreRefPointObj>"
  Bus Selector1/3 -> Product3/2  "<cosCoef>"
  Bus Selector1/2 -> Product3/1  "<ObjWidthCorr>"
  Product3/1 -> Gain1/1
  Add1/1 -> dyPreRLObj/1
  Add/1 -> dxPreRLObj/1
  Bus Selector/1 -> Add/1  "<dxPreRefPointObj>"
  Product1/1 -> Gain/1
  Bus Selector/3 -> Product1/2  "<sinCoef>"
  Bus Selector/2 -> Product1/1  "<ObjWidthCorr>"
  inputSignals/1 -> Bus Selector1/1, Bus Selector/1
  Gain/1 -> Add/2
  Gain1/1 -> Add1/2

== system TosAeb/FcwAebJa_Step_sys/ALG_AEBSystem/ALG_AEBController/AEB_JaObjCalcApp/JAVehTgtInfoCalc/AEBJAVehObjCalc/JAVehObjInfoCalc/ObjCrossFlgCal/CollisionProbCalc/Subsystem/ObjBoundingBoxRear/ObjRUCoordinate ==
  inputSignals : Inport
  Add : Sum  Inputs=+++  OutDataTypeStr=single
  Add1 : Sum  Inputs=++-  OutDataTypeStr=single
  Bus Selector : BusSelector  OutputSignals=dxPreRefPointObj,ObjLengthCorr,sincosCoef.cosCoef,ObjWidthCorr,sincosCoef.sinCoef
  Bus Selector1 : BusSelector  OutputSignals=dyPreRefPointObj,ObjLengthCorr,sincosCoef.sinCoef,ObjWidthCorr,sincosCoef.cosCoef
  Gain : Gain  Gain=0.5  OutDataTypeStr=single
  Gain1 : Gain  Gain=0.5  OutDataTypeStr=single
  Product{,1..3} (4 alike) : Product  OutDataTypeStr=single
  dxPreRUObj : Outport
  dyPreRUObj : Outport  Port=2
  wires:
  inputSignals/1 -> Bus Selector1/1, Bus Selector/1
  Bus Selector/2 -> Product/1  "<ObjLengthCorr>"
  Bus Selector/3 -> Product/2  "<cosCoef>"
  Bus Selector/4 -> Product1/1  "<ObjWidthCorr>"
  Bus Selector/5 -> Product1/2  "<sinCoef>"
  Product1/1 -> Gain1/1
  Bus Selector/1 -> Add/1  "<dxPreRefPointObj>"
  Product/1 -> Add/2
  Add/1 -> dxPreRUObj/1
  Add1/1 -> dyPreRUObj/1
  Product2/1 -> Add1/2
  Product3/1 -> Gain/1
  Bus Selector1/2 -> Product2/1  "<ObjLengthCorr>"
  Bus Selector1/4 -> Product3/1  "<ObjWidthCorr>"
  Bus Selector1/3 -> Product2/2  "<sinCoef>"
  Bus Selector1/5 -> Product3/2  "<cosCoef>"
  Bus Selector1/1 -> Add1/1  "<dyPreRefPointObj>"
  Gain/1 -> Add1/3
  Gain1/1 -> Add/3

== system TosAeb/FcwAebJa_Step_sys/ALG_AEBSystem/ALG_AEBController/AEB_JaObjCalcApp/JAVehTgtInfoCalc/AEBJAVehObjCalc/JAVehObjInfoCalc/ObjCrossFlgCal/CollisionProbCalc/Subsystem/ObjBoundingBoxfront/ObjLLCoordinate ==
  inputSignals : Inport
  Add : Sum  OutDataTypeStr=single
  Add1 : Sum  Inputs=+-  OutDataTypeStr=single
  Bus Selector : BusSelector  OutputSignals=dxPreRefPointObj,ObjWidthCorr,sincosCoef.sinCoef
  Bus Selector1 : BusSelector  OutputSignals=dyPreRefPointObj,ObjWidthCorr,sincosCoef.cosCoef
  Gain : Gain  Gain=0.5  OutDataTypeStr=single
  Gain1 : Gain  Gain=0.5  OutDataTypeStr=single
  Product1 : Product  OutDataTypeStr=single
  Product3 : Product  OutDataTypeStr=single
  dxPre_LLObj : Outport
  dyPreLLObj : Outport  Port=2
  wires:
  Bus Selector1/1 -> Add1/1  "<dyPreRefPointObj>"
  Bus Selector1/3 -> Product3/2  "<cosCoef>"
  Bus Selector1/2 -> Product3/1  "<ObjWidthCorr>"
  Product3/1 -> Gain/1
  Add1/1 -> dyPreLLObj/1
  Add/1 -> dxPre_LLObj/1
  Bus Selector/1 -> Add/1  "<dxPreRefPointObj>"
  Product1/1 -> Gain1/1
  Bus Selector/3 -> Product1/2  "<sinCoef>"
  Bus Selector/2 -> Product1/1  "<ObjWidthCorr>"
  inputSignals/1 -> Bus Selector1/1, Bus Selector/1
  Gain/1 -> Add1/2
  Gain1/1 -> Add/2

== system TosAeb/FcwAebJa_Step_sys/ALG_AEBSystem/ALG_AEBController/AEB_JaObjCalcApp/JAVehTgtInfoCalc/AEBJAVehObjCalc/JAVehObjInfoCalc/ObjCrossFlgCal/CollisionProbCalc/Subsystem/ObjBoundingBoxfront/ObjLUCoordinate ==
  inputSignals : Inport
  Add : Sum  Inputs=+-+  OutDataTypeStr=single
  Add1 : Sum  Inputs=+--  OutDataTypeStr=single
  Bus Selector : BusSelector  OutputSignals=dxPreRefPointObj,ObjLengthCorr,sincosCoef.cosCoef,ObjWidthCorr,sincosCoef.sinCoef
  Bus Selector1 : BusSelector  OutputSignals=dyPreRefPointObj,ObjLengthCorr,sincosCoef.sinCoef,ObjWidthCorr,sincosCoef.cosCoef
  Gain : Gain  Gain=0.5  OutDataTypeStr=single
  Gain1 : Gain  Gain=0.5  OutDataTypeStr=single
  Product{,1..3} (4 alike) : Product  OutDataTypeStr=single
  dxPreLUObj : Outport
  dyPreLUobj : Outport  Port=2
  wires:
  inputSignals/1 -> Bus Selector1/1, Bus Selector/1
  Bus Selector/2 -> Product/1  "<ObjLengthCorr>"
  Bus Selector/3 -> Product/2  "<cosCoef>"
  Bus Selector/4 -> Product1/1  "<ObjWidthCorr>"
  Bus Selector/5 -> Product1/2  "<sinCoef>"
  Product1/1 -> Gain1/1
  Bus Selector/1 -> Add/1  "<dxPreRefPointObj>"
  Product/1 -> Add/2
  Add/1 -> dxPreLUObj/1
  Add1/1 -> dyPreLUobj/1
  Product2/1 -> Add1/2
  Product3/1 -> Gain/1
  Bus Selector1/2 -> Product2/1  "<ObjLengthCorr>"
  Bus Selector1/4 -> Product3/1  "<ObjWidthCorr>"
  Bus Selector1/3 -> Product2/2  "<sinCoef>"
  Bus Selector1/5 -> Product3/2  "<cosCoef>"
  Bus Selector1/1 -> Add1/1  "<dyPreRefPointObj>"
  Gain/1 -> Add1/3
  Gain1/1 -> Add/3

== system TosAeb/FcwAebJa_Step_sys/ALG_AEBSystem/ALG_AEBController/AEB_JaObjCalcApp/JAVehTgtInfoCalc/AEBJAVehObjCalc/JAVehObjInfoCalc/ObjCrossFlgCal/CollisionProbCalc/Subsystem/ObjBoundingBoxfront/ObjRLCoordinate ==
  inputSignals : Inport
  Add : Sum  Inputs=+-  OutDataTypeStr=single
  Add1 : Sum  OutDataTypeStr=single
  Bus Selector : BusSelector  OutputSignals=dxPreRefPointObj,ObjWidthCorr,sincosCoef.sinCoef
  Bus Selector1 : BusSelector  OutputSignals=dyPreRefPointObj,ObjWidthCorr,sincosCoef.cosCoef
  Gain : Gain  Gain=0.5  OutDataTypeStr=single
  Gain1 : Gain  Gain=0.5  OutDataTypeStr=single
  Product1 : Product  OutDataTypeStr=single
  Product3 : Product  OutDataTypeStr=single
  dxPreRLObj : Outport
  dyPreRLObj : Outport  Port=2
  wires:
  Bus Selector1/1 -> Add1/1  "<dyPreRefPointObj>"
  Bus Selector1/3 -> Product3/2  "<cosCoef>"
  Bus Selector1/2 -> Product3/1  "<ObjWidthCorr>"
  Product3/1 -> Gain1/1
  Add1/1 -> dyPreRLObj/1
  Add/1 -> dxPreRLObj/1
  Bus Selector/1 -> Add/1  "<dxPreRefPointObj>"
  Product1/1 -> Gain/1
  Bus Selector/3 -> Product1/2  "<sinCoef>"
  Bus Selector/2 -> Product1/1  "<ObjWidthCorr>"
  inputSignals/1 -> Bus Selector1/1, Bus Selector/1
  Gain/1 -> Add/2
  Gain1/1 -> Add1/2
